simulink model "All_64_Coils"  (R2021b)
settings: Solver=FixedStepAuto  StartTime=-3000e-6  StopTime=3000e-6  FixedStep=25e-6  RelTol=1e-3
(part 2 of 70)

== system All_64_Coils/Coil-51 ==
  x : Inport
  y : Inport  Port=2
  z : Inport  Port=3
  Current : Inport  Port=4
  Add{,1..4} (5 alike) : Sum
  Display{,1..3} (4 alike) : Display
  Inner_5_coils : Subsystem
  Outer_5_Coils : Subsystem
  Scope : Scope  NumInputPorts=4
  Scope1 : Scope
  wires:
  Outer_5_Coils/3(Output2) -> Add2/2
  Inner_5_coils/2(Output1) -> Add1/1
  Outer_5_Coils/4(Output3) -> Add3/2
  z/1 -> Outer_5_Coils/3(Input2), Inner_5_coils/3(Input2)
  Add/1 -> Scope/1, Display/1  "Fxx"
  y/1 -> Outer_5_Coils/2(Input1), Inner_5_coils/2(Input1)
  Inner_5_coils/4(Output3) -> Add3/1
  Inner_5_coils/1(Output) -> Add/1
  Add3/1 -> Scope/4, Display3/1, Add4/2  "Fyz"
  Add1/1 -> Scope/2, Display1/1, Add4/1  "Fxz"
  Outer_5_Coils/1(Output) -> Add/2
  x/1 -> Outer_5_Coils/1(Input), Inner_5_coils/1(Input)
  Add2/1 -> Scope/3, Display2/1  "Fyy"
  Outer_5_Coils/2(Output1) -> Add1/2
  Current/1 -> Outer_5_Coils/4(Input3), Inner_5_coils/4(Input3)
  Inner_5_coils/3(Output2) -> Add2/1
  Add4/1 -> Scope1/1  "Total_Force"

== system All_64_Coils/Coil-52 ==
  x : Inport
  y : Inport  Port=2
  z : Inport  Port=3
  Current : Inport  Port=4
  Add{,1..4} (5 alike) : Sum
  Display{,1..3} (4 alike) : Display
  Inner_5_coils : Subsystem
  Outer_5_Coils : Subsystem
  Scope : Scope  NumInputPorts=4
  Scope1 : Scope
  wires:
  Add4/1 -> Scope1/1  "Total_Force"
  Inner_5_coils/3(Output2) -> Add2/1
  Current/1 -> Inner_5_coils/4(Input3), Outer_5_Coils/4(Input3)
  Outer_5_Coils/2(Output1) -> Add1/2
  Add2/1 -> Display2/1, Scope/3  "Fyy"
  x/1 -> Inner_5_coils/1(Input), Outer_5_Coils/1(Input)
  Outer_5_Coils/1(Output) -> Add/2
  Add1/1 -> Add4/1, Display1/1, Scope/2  "Fxz"
  Add3/1 -> Add4/2, Display3/1, Scope/4  "Fyz"
  Inner_5_coils/1(Output) -> Add/1
  Inner_5_coils/4(Output3) -> Add3/1
  y/1 -> Inner_5_coils/2(Input1), Outer_5_Coils/2(Input1)
  Add/1 -> Display/1, Scope/1  "Fxx"
  z/1 -> Inner_5_coils/3(Input2), Outer_5_Coils/3(Input2)
  Outer_5_Coils/4(Output3) -> Add3/2
  Inner_5_coils/2(Output1) -> Add1/1
  Outer_5_Coils/3(Output2) -> Add2/2

== system All_64_Coils/Coil-53 ==
  x : Inport
  y : Inport  Port=2
  z : Inport  Port=3
  Current : Inport  Port=4
  Add{,1..4} (5 alike) : Sum
  Display{,1..3} (4 alike) : Display
  Inner_5_coils : Subsystem
  Outer_5_Coils : Subsystem
  Scope : Scope  NumInputPorts=4
  Scope1 : Scope
  wires:
  Outer_5_Coils/3(Output2) -> Add2/2
  Inner_5_coils/2(Output1) -> Add1/1
  Outer_5_Coils/4(Output3) -> Add3/2
  z/1 -> Outer_5_Coils/3(Input2), Inner_5_coils/3(Input2)
  Add/1 -> Scope/1, Display/1  "Fxx"
  y/1 -> Outer_5_Coils/2(Input1), Inner_5_coils/2(Input1)
  Inner_5_coils/4(Output3) -> Add3/1
  Inner_5_coils/1(Output) -> Add/1
  Add3/1 -> Scope/4, Display3/1, Add4/2  "Fyz"
  Add1/1 -> Scope/2, Display1/1, Add4/1  "Fxz"
  Outer_5_Coils/1(Output) -> Add/2
  x/1 -> Outer_5_Coils/1(Input), Inner_5_coils/1(Input)
  Add2/1 -> Scope/3, Display2/1  "Fyy"
  Outer_5_Coils/2(Output1) -> Add1/2
  Current/1 -> Outer_5_Coils/4(Input3), Inner_5_coils/4(Input3)
  Inner_5_coils/3(Output2) -> Add2/1
  Add4/1 -> Scope1/1  "Total_Force"

== system All_64_Coils/Coil-54 ==
  x : Inport
  y : Inport  Port=2
  z : Inport  Port=3
  Current : Inport  Port=4
  Add{,1..4} (5 alike) : Sum
  Display{,1..3} (4 alike) : Display
  Inner_5_coils : Subsystem
  Outer_5_Coils : Subsystem
  Scope : Scope  NumInputPorts=4
  Scope1 : Scope
  wires:
  Add4/1 -> Scope1/1  "Total_Force"
  Inner_5_coils/3(Output2) -> Add2/1
  Current/1 -> Inner_5_coils/4(Input3), Outer_5_Coils/4(Input3)
  Outer_5_Coils/2(Output1) -> Add1/2
  Add2/1 -> Display2/1, Scope/3  "Fyy"
  x/1 -> Inner_5_coils/1(Input), Outer_5_Coils/1(Input)
  Outer_5_Coils/1(Output) -> Add/2
  Add1/1 -> Add4/1, Display1/1, Scope/2  "Fxz"
  Add3/1 -> Add4/2, Display3/1, Scope/4  "Fyz"
  Inner_5_coils/1(Output) -> Add/1
  Inner_5_coils/4(Output3) -> Add3/1
  y/1 -> Inner_5_coils/2(Input1), Outer_5_Coils/2(Input1)
  Add/1 -> Display/1, Scope/1  "Fxx"
  z/1 -> Inner_5_coils/3(Input2), Outer_5_Coils/3(Input2)
  Outer_5_Coils/4(Output3) -> Add3/2
  Inner_5_coils/2(Output1) -> Add1/1
  Outer_5_Coils/3(Output2) -> Add2/2

== system All_64_Coils/Coil-55 ==
  x : Inport
  y : Inport  Port=2
  z : Inport  Port=3
  Current : Inport  Port=4
  Add{,1..4} (5 alike) : Sum
  Display{,1..3} (4 alike) : Display
  Inner_5_coils : Subsystem
  Outer_5_Coils : Subsystem
  Scope : Scope  NumInputPorts=4
  Scope1 : Scope
  wires:
  Add4/1 -> Scope1/1  "Total_Force"
  Inner_5_coils/3(Output2) -> Add2/1
  Current/1 -> Inner_5_coils/4(Input3), Outer_5_Coils/4(Input3)
  Outer_5_Coils/2(Output1) -> Add1/2
  Add2/1 -> Display2/1, Scope/3  "Fyy"
  x/1 -> Inner_5_coils/1(Input), Outer_5_Coils/1(Input)
  Outer_5_Coils/1(Output) -> Add/2
  Add1/1 -> Add4/1, Display1/1, Scope/2  "Fxz"
  Add3/1 -> Add4/2, Display3/1, Scope/4  "Fyz"
  Inner_5_coils/1(Output) -> Add/1
  Inner_5_coils/4(Output3) -> Add3/1
  y/1 -> Inner_5_coils/2(Input1), Outer_5_Coils/2(Input1)
  Add/1 -> Display/1, Scope/1  "Fxx"
  z/1 -> Inner_5_coils/3(Input2), Outer_5_Coils/3(Input2)
  Outer_5_Coils/4(Output3) -> Add3/2
  Inner_5_coils/2(Output1) -> Add1/1
  Outer_5_Coils/3(Output2) -> Add2/2

== system All_64_Coils/Coil-56 ==
  x : Inport
  y : Inport  Port=2
  z : Inport  Port=3
  Current : Inport  Port=4
  Add{,1..4} (5 alike) : Sum
  Display{,1..3} (4 alike) : Display
  Inner_5_coils : Subsystem
  Outer_5_Coils : Subsystem
  Scope : Scope  NumInputPorts=4
  Scope1 : Scope
  wires:
  Add4/1 -> Scope1/1  "Total_Force"
  Inner_5_coils/3(Output2) -> Add2/1
  Current/1 -> Inner_5_coils/4(Input3), Outer_5_Coils/4(Input3)
  Outer_5_Coils/2(Output1) -> Add1/2
  Add2/1 -> Display2/1, Scope/3  "Fyy"
  x/1 -> Inner_5_coils/1(Input), Outer_5_Coils/1(Input)
  Outer_5_Coils/1(Output) -> Add/2
  Add1/1 -> Add4/1, Display1/1, Scope/2  "Fxz"
  Add3/1 -> Add4/2, Display3/1, Scope/4  "Fyz"
  Inner_5_coils/1(Output) -> Add/1
  Inner_5_coils/4(Output3) -> Add3/1
  y/1 -> Inner_5_coils/2(Input1), Outer_5_Coils/2(Input1)
  Add/1 -> Display/1, Scope/1  "Fxx"
  z/1 -> Inner_5_coils/3(Input2), Outer_5_Coils/3(Input2)
  Outer_5_Coils/4(Output3) -> Add3/2
  Inner_5_coils/2(Output1) -> Add1/1
  Outer_5_Coils/3(Output2) -> Add2/2

== system All_64_Coils/Coil-57 ==
  x : Inport
  y : Inport  Port=2
  z : Inport  Port=3
  Current : Inport  Port=4
  Add{,1..4} (5 alike) : Sum
  Display{,1..3} (4 alike) : Display
  Inner_5_coils : Subsystem
  Outer_5_Coils : Subsystem
  Scope : Scope  NumInputPorts=4
  Scope1 : Scope
  wires:
  Outer_5_Coils/3(Output2) -> Add2/2
  Inner_5_coils/2(Output1) -> Add1/1
  Outer_5_Coils/4(Output3) -> Add3/2
  z/1 -> Outer_5_Coils/3(Input2), Inner_5_coils/3(Input2)
  Add/1 -> Scope/1, Display/1  "Fxx"
  y/1 -> Outer_5_Coils/2(Input1), Inner_5_coils/2(Input1)
  Inner_5_coils/4(Output3) -> Add3/1
  Inner_5_coils/1(Output) -> Add/1
  Add3/1 -> Scope/4, Display3/1, Add4/2  "Fyz"
  Add1/1 -> Scope/2, Display1/1, Add4/1  "Fxz"
  Outer_5_Coils/1(Output) -> Add/2
  x/1 -> Outer_5_Coils/1(Input), Inner_5_coils/1(Input)
  Add2/1 -> Scope/3, Display2/1  "Fyy"
  Outer_5_Coils/2(Output1) -> Add1/2
  Current/1 -> Outer_5_Coils/4(Input3), Inner_5_coils/4(Input3)
  Inner_5_coils/3(Output2) -> Add2/1
  Add4/1 -> Scope1/1  "Total_Force"

== system All_64_Coils/Coil-58 ==
  x : Inport
  y : Inport  Port=2
  z : Inport  Port=3
  Current : Inport  Port=4
  Add{,1..4} (5 alike) : Sum
  Display{,1..3} (4 alike) : Display
  Inner_5_coils : Subsystem
  Outer_5_Coils : Subsystem
  Scope : Scope  NumInputPorts=4
  Scope1 : Scope
  wires:
  Outer_5_Coils/3(Output2) -> Add2/2
  Inner_5_coils/2(Output1) -> Add1/1
  Outer_5_Coils/4(Output3) -> Add3/2
  z/1 -> Outer_5_Coils/3(Input2), Inner_5_coils/3(Input2)
  Add/1 -> Scope/1, Display/1  "Fxx"
  y/1 -> Outer_5_Coils/2(Input1), Inner_5_coils/2(Input1)
  Inner_5_coils/4(Output3) -> Add3/1
  Inner_5_coils/1(Output) -> Add/1
  Add3/1 -> Scope/4, Display3/1, Add4/2  "Fyz"
  Add1/1 -> Scope/2, Display1/1, Add4/1  "Fxz"
  Outer_5_Coils/1(Output) -> Add/2
  x/1 -> Outer_5_Coils/1(Input), Inner_5_coils/1(Input)
  Add2/1 -> Scope/3, Display2/1  "Fyy"
  Outer_5_Coils/2(Output1) -> Add1/2
  Current/1 -> Outer_5_Coils/4(Input3), Inner_5_coils/4(Input3)
  Inner_5_coils/3(Output2) -> Add2/1
  Add4/1 -> Scope1/1  "Total_Force"

== system All_64_Coils/Coil-59 ==
  x : Inport
  y : Inport  Port=2
  z : Inport  Port=3
  Current : Inport  Port=4
  Add{,1..4} (5 alike) : Sum
  Display{,1..3} (4 alike) : Display
  Inner_5_coils : Subsystem
  Outer_5_Coils : Subsystem
  Scope : Scope  NumInputPorts=4
  Scope1 : Scope
  wires:
  Outer_5_Coils/3(Output2) -> Add2/2
  Inner_5_coils/2(Output1) -> Add1/1
  Outer_5_Coils/4(Output3) -> Add3/2
  z/1 -> Outer_5_Coils/3(Input2), Inner_5_coils/3(Input2)
  Add/1 -> Scope/1, Display/1  "Fxx"
  y/1 -> Outer_5_Coils/2(Input1), Inner_5_coils/2(Input1)
  Inner_5_coils/4(Output3) -> Add3/1
  Inner_5_coils/1(Output) -> Add/1
  Add3/1 -> Scope/4, Display3/1, Add4/2  "Fyz"
  Add1/1 -> Scope/2, Display1/1, Add4/1  "Fxz"
  Outer_5_Coils/1(Output) -> Add/2
  x/1 -> Outer_5_Coils/1(Input), Inner_5_coils/1(Input)
  Add2/1 -> Scope/3, Display2/1  "Fyy"
  Outer_5_Coils/2(Output1) -> Add1/2
  Current/1 -> Outer_5_Coils/4(Input3), Inner_5_coils/4(Input3)
  Inner_5_coils/3(Output2) -> Add2/1
  Add4/1 -> Scope1/1  "Total_Force"

== system All_64_Coils/Coil-6 ==
  x : Inport
  y : Inport  Port=2
  z : Inport  Port=3
  Current : Inport  Port=4
  Add{,1..4} (5 alike) : Sum
  Display{,1..3} (4 alike) : Display
  Inner_5_coils : Subsystem
  Outer_5_Coils : Subsystem
  Scope : Scope  NumInputPorts=4
  Scope1 : Scope
  wires:
  Add4/1 -> Scope1/1  "Total_Force"
  Inner_5_coils/3(Output2) -> Add2/1
  Current/1 -> Inner_5_coils/4(Input3), Outer_5_Coils/4(Input3)
  Outer_5_Coils/2(Output1) -> Add1/2
  Add2/1 -> Display2/1, Scope/3  "Fyy"
  x/1 -> Inner_5_coils/1(Input), Outer_5_Coils/1(Input)
  Outer_5_Coils/1(Output) -> Add/2
  Add1/1 -> Add4/1, Display1/1, Scope/2  "Fxz"
  Add3/1 -> Add4/2, Display3/1, Scope/4  "Fyz"
  Inner_5_coils/1(Output) -> Add/1
  Inner_5_coils/4(Output3) -> Add3/1
  y/1 -> Inner_5_coils/2(Input1), Outer_5_Coils/2(Input1)
  Add/1 -> Display/1, Scope/1  "Fxx"
  z/1 -> Inner_5_coils/3(Input2), Outer_5_Coils/3(Input2)
  Outer_5_Coils/4(Output3) -> Add3/2
  Inner_5_coils/2(Output1) -> Add1/1
  Outer_5_Coils/3(Output2) -> Add2/2

== system All_64_Coils/Coil-60 ==
  x : Inport
  y : Inport  Port=2
  z : Inport  Port=3
  Current : Inport  Port=4
  Add{,1..4} (5 alike) : Sum
  Display{,1..3} (4 alike) : Display
  Inner_5_coils : Subsystem
  Outer_5_Coils : Subsystem
  Scope : Scope  NumInputPorts=4
  Scope1 : Scope
  wires:
  Add4/1 -> Scope1/1  "Total_Force"
  Inner_5_coils/3(Output2) -> Add2/1
  Current/1 -> Inner_5_coils/4(Input3), Outer_5_Coils/4(Input3)
  Outer_5_Coils/2(Output1) -> Add1/2
  Add2/1 -> Display2/1, Scope/3  "Fyy"
  x/1 -> Inner_5_coils/1(Input), Outer_5_Coils/1(Input)
  Outer_5_Coils/1(Output) -> Add/2
  Add1/1 -> Add4/1, Display1/1, Scope/2  "Fxz"
  Add3/1 -> Add4/2, Display3/1, Scope/4  "Fyz"
  Inner_5_coils/1(Output) -> Add/1
  Inner_5_coils/4(Output3) -> Add3/1
  y/1 -> Inner_5_coils/2(Input1), Outer_5_Coils/2(Input1)
  Add/1 -> Display/1, Scope/1  "Fxx"
  z/1 -> Inner_5_coils/3(Input2), Outer_5_Coils/3(Input2)
  Outer_5_Coils/4(Output3) -> Add3/2
  Inner_5_coils/2(Output1) -> Add1/1
  Outer_5_Coils/3(Output2) -> Add2/2

== system All_64_Coils/Coil-61 ==
  x : Inport
  y : Inport  Port=2
  z : Inport  Port=3
  Current : Inport  Port=4
  Add{,1..4} (5 alike) : Sum
  Display{,1..3} (4 alike) : Display
  Inner_5_coils : Subsystem
  Outer_5_Coils : Subsystem
  Scope : Scope  NumInputPorts=4
  Scope1 : Scope
  wires:
  Outer_5_Coils/3(Output2) -> Add2/2
  Inner_5_coils/2(Output1) -> Add1/1
  Outer_5_Coils/4(Output3) -> Add3/2
  z/1 -> Outer_5_Coils/3(Input2), Inner_5_coils/3(Input2)
  Add/1 -> Scope/1, Display/1  "Fxx"
  y/1 -> Outer_5_Coils/2(Input1), Inner_5_coils/2(Input1)
  Inner_5_coils/4(Output3) -> Add3/1
  Inner_5_coils/1(Output) -> Add/1
  Add3/1 -> Scope/4, Display3/1, Add4/2  "Fyz"
  Add1/1 -> Scope/2, Display1/1, Add4/1  "Fxz"
  Outer_5_Coils/1(Output) -> Add/2
  x/1 -> Outer_5_Coils/1(Input), Inner_5_coils/1(Input)
  Add2/1 -> Scope/3, Display2/1  "Fyy"
  Outer_5_Coils/2(Output1) -> Add1/2
  Current/1 -> Outer_5_Coils/4(Input3), Inner_5_coils/4(Input3)
  Inner_5_coils/3(Output2) -> Add2/1
  Add4/1 -> Scope1/1  "Total_Force"

== system All_64_Coils/Coil-62 ==
  x : Inport
  y : Inport  Port=2
  z : Inport  Port=3
  Current : Inport  Port=4
  Add{,1..4} (5 alike) : Sum
  Display{,1..3} (4 alike) : Display
  Inner_5_coils : Subsystem
  Outer_5_Coils : Subsystem
  Scope : Scope  NumInputPorts=4
  Scope1 : Scope
  wires:
  Outer_5_Coils/3(Output2) -> Add2/2
  Inner_5_coils/2(Output1) -> Add1/1
  Outer_5_Coils/4(Output3) -> Add3/2
  z/1 -> Outer_5_Coils/3(Input2), Inner_5_coils/3(Input2)
  Add/1 -> Scope/1, Display/1  "Fxx"
  y/1 -> Outer_5_Coils/2(Input1), Inner_5_coils/2(Input1)
  Inner_5_coils/4(Output3) -> Add3/1
  Inner_5_coils/1(Output) -> Add/1
  Add3/1 -> Scope/4, Display3/1, Add4/2  "Fyz"
  Add1/1 -> Scope/2, Display1/1, Add4/1  "Fxz"
  Outer_5_Coils/1(Output) -> Add/2
  x/1 -> Outer_5_Coils/1(Input), Inner_5_coils/1(Input)
  Add2/1 -> Scope/3, Display2/1  "Fyy"
  Outer_5_Coils/2(Output1) -> Add1/2
  Current/1 -> Outer_5_Coils/4(Input3), Inner_5_coils/4(Input3)
  Inner_5_coils/3(Output2) -> Add2/1
  Add4/1 -> Scope1/1  "Total_Force"

== system All_64_Coils/Coil-63 ==
  x : Inport
  y : Inport  Port=2
  z : Inport  Port=3
  Current : Inport  Port=4
  Add{,1..4} (5 alike) : Sum
  Display{,1..3} (4 alike) : Display
  Inner_5_coils : Subsystem
  Outer_5_Coils : Subsystem
  Scope : Scope  NumInputPorts=4
  Scope1 : Scope
  wires:
  Add4/1 -> Scope1/1  "Total_Force"
  Inner_5_coils/3(Output2) -> Add2/1
  Current/1 -> Inner_5_coils/4(Input3), Outer_5_Coils/4(Input3)
  Outer_5_Coils/2(Output1) -> Add1/2
  Add2/1 -> Display2/1, Scope/3  "Fyy"
  x/1 -> Inner_5_coils/1(Input), Outer_5_Coils/1(Input)
  Outer_5_Coils/1(Output) -> Add/2
  Add1/1 -> Add4/1, Display1/1, Scope/2  "Fxz"
  Add3/1 -> Add4/2, Display3/1, Scope/4  "Fyz"
  Inner_5_coils/1(Output) -> Add/1
  Inner_5_coils/4(Output3) -> Add3/1
  y/1 -> Inner_5_coils/2(Input1), Outer_5_Coils/2(Input1)
  Add/1 -> Display/1, Scope/1  "Fxx"
  z/1 -> Inner_5_coils/3(Input2), Outer_5_Coils/3(Input2)
  Outer_5_Coils/4(Output3) -> Add3/2
  Inner_5_coils/2(Output1) -> Add1/1
  Outer_5_Coils/3(Output2) -> Add2/2

== system All_64_Coils/Coil-64 ==
  x : Inport
  y : Inport  Port=2
  z : Inport  Port=3
  Current : Inport  Port=4
  Add{,1..4} (5 alike) : Sum
  Display{,1..3} (4 alike) : Display
  Inner_5_coils : Subsystem
  Outer_5_Coils : Subsystem
  Scope : Scope  NumInputPorts=4
  Scope1 : Scope
  wires:
  Add4/1 -> Scope1/1  "Total_Force"
  Inner_5_coils/3(Output2) -> Add2/1
  Current/1 -> Inner_5_coils/4(Input3), Outer_5_Coils/4(Input3)
  Outer_5_Coils/2(Output1) -> Add1/2
  Add2/1 -> Display2/1, Scope/3  "Fyy"
  x/1 -> Inner_5_coils/1(Input), Outer_5_Coils/1(Input)
  Outer_5_Coils/1(Output) -> Add/2
  Add1/1 -> Add4/1, Display1/1, Scope/2  "Fxz"
  Add3/1 -> Add4/2, Display3/1, Scope/4  "Fyz"
  Inner_5_coils/1(Output) -> Add/1
  Inner_5_coils/4(Output3) -> Add3/1
  y/1 -> Inner_5_coils/2(Input1), Outer_5_Coils/2(Input1)
  Add/1 -> Display/1, Scope/1  "Fxx"
  z/1 -> Inner_5_coils/3(Input2), Outer_5_Coils/3(Input2)
  Outer_5_Coils/4(Output3) -> Add3/2
  Inner_5_coils/2(Output1) -> Add1/1
  Outer_5_Coils/3(Output2) -> Add2/2

== system All_64_Coils/Coil-7 ==
  x : Inport
  y : Inport  Port=2
  z : Inport  Port=3
  Current : Inport  Port=4
  Add{,1..4} (5 alike) : Sum
  Display{,1..3} (4 alike) : Display
  Inner_5_coils : Subsystem
  Outer_5_Coils : Subsystem
  Scope : Scope  NumInputPorts=4
  Scope1 : Scope
  wires:
  Add4/1 -> Scope1/1  "Total_Force"
  Inner_5_coils/3(Output2) -> Add2/1
  Current/1 -> Inner_5_coils/4(Input3), Outer_5_Coils/4(Input3)
  Outer_5_Coils/2(Output1) -> Add1/2
  Add2/1 -> Display2/1, Scope/3  "Fyy"
  x/1 -> Inner_5_coils/1(Input), Outer_5_Coils/1(Input)
  Outer_5_Coils/1(Output) -> Add/2
  Add1/1 -> Add4/1, Display1/1, Scope/2  "Fxz"
  Add3/1 -> Add4/2, Display3/1, Scope/4  "Fyz"
  Inner_5_coils/1(Output) -> Add/1
  Inner_5_coils/4(Output3) -> Add3/1
  y/1 -> Inner_5_coils/2(Input1), Outer_5_Coils/2(Input1)
  Add/1 -> Display/1, Scope/1  "Fxx"
  z/1 -> Inner_5_coils/3(Input2), Outer_5_Coils/3(Input2)
  Outer_5_Coils/4(Output3) -> Add3/2
  Inner_5_coils/2(Output1) -> Add1/1
  Outer_5_Coils/3(Output2) -> Add2/2

== system All_64_Coils/Coil-8 ==
  x : Inport
  y : Inport  Port=2
  z : Inport  Port=3
  Current : Inport  Port=4
  Add{,1..4} (5 alike) : Sum
  Display{,1..3} (4 alike) : Display
  Inner_5_coils : Subsystem
  Outer_5_Coils : Subsystem
  Scope : Scope  NumInputPorts=4
  Scope1 : Scope
  wires:
  Outer_5_Coils/3(Output2) -> Add2/2
  Inner_5_coils/2(Output1) -> Add1/1
  Outer_5_Coils/4(Output3) -> Add3/2
  z/1 -> Outer_5_Coils/3(Input2), Inner_5_coils/3(Input2)
  Add/1 -> Scope/1, Display/1  "Fxx"
  y/1 -> Outer_5_Coils/2(Input1), Inner_5_coils/2(Input1)
  Inner_5_coils/4(Output3) -> Add3/1
  Inner_5_coils/1(Output) -> Add/1
  Add3/1 -> Scope/4, Display3/1, Add4/2  "Fyz"
  Add1/1 -> Scope/2, Display1/1, Add4/1  "Fxz"
  Outer_5_Coils/1(Output) -> Add/2
  x/1 -> Outer_5_Coils/1(Input), Inner_5_coils/1(Input)
  Add2/1 -> Scope/3, Display2/1  "Fyy"
  Outer_5_Coils/2(Output1) -> Add1/2
  Current/1 -> Outer_5_Coils/4(Input3), Inner_5_coils/4(Input3)
  Inner_5_coils/3(Output2) -> Add2/1
  Add4/1 -> Scope1/1  "Total_Force"

== system All_64_Coils/Coil-9 ==
  x : Inport
  y : Inport  Port=2
  z : Inport  Port=3
  Current : Inport  Port=4
  Add{,1..4} (5 alike) : Sum
  Display{,1..3} (4 alike) : Display
  Inner_5_coils : Subsystem
  Outer_5_Coils : Subsystem
  Scope : Scope  NumInputPorts=4
  Scope1 : Scope
  wires:
  Outer_5_Coils/3(Output2) -> Add2/2
  Inner_5_coils/2(Output1) -> Add1/1
  Outer_5_Coils/4(Output3) -> Add3/2
  z/1 -> Outer_5_Coils/3(Input2), Inner_5_coils/3(Input2)
  Add/1 -> Scope/1, Display/1  "Fxx"
  y/1 -> Outer_5_Coils/2(Input1), Inner_5_coils/2(Input1)
  Inner_5_coils/4(Output3) -> Add3/1
  Inner_5_coils/1(Output) -> Add/1
  Add3/1 -> Scope/4, Display3/1, Add4/2  "Fyz"
  Add1/1 -> Scope/2, Display1/1, Add4/1  "Fxz"
  Outer_5_Coils/1(Output) -> Add/2
  x/1 -> Outer_5_Coils/1(Input), Inner_5_coils/1(Input)
  Add2/1 -> Scope/3, Display2/1  "Fyy"
  Outer_5_Coils/2(Output1) -> Add1/2
  Current/1 -> Outer_5_Coils/4(Input3), Inner_5_coils/4(Input3)
  Inner_5_coils/3(Output2) -> Add2/1
  Add4/1 -> Scope1/1  "Total_Force"

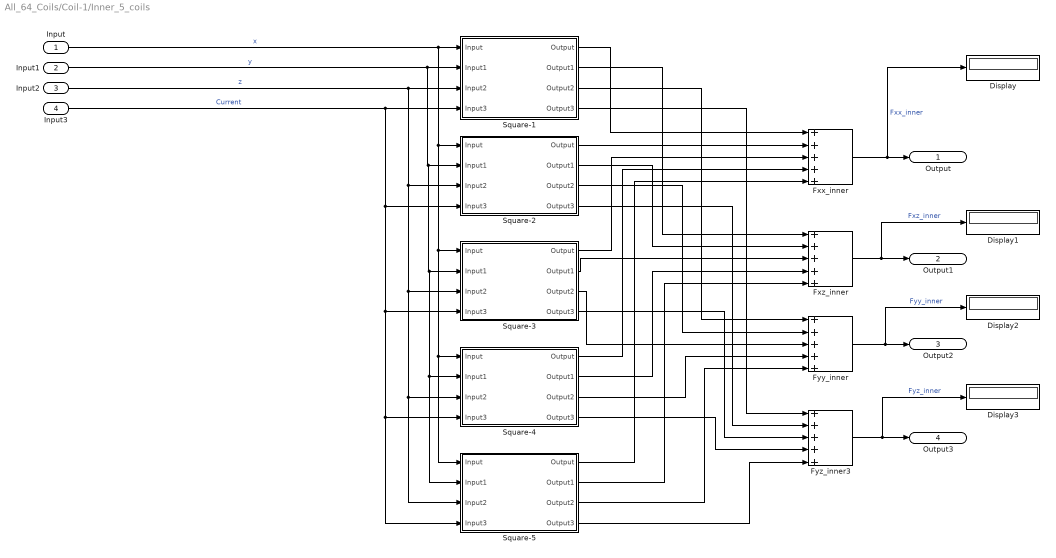
== system All_64_Coils/Coil-1/Inner_5_coils ==
  Input : Inport
  Input1 : Inport  Port=2
  Input2 : Inport  Port=3
  Input3 : Inport  Port=4
  Display{,1..3} (4 alike) : Display
  Fxx_inner : Sum  Inputs=+++++
  Fxz_inner : Sum  Inputs=+++++
  Fyy_inner : Sum  Inputs=+++++
  Fyz_inner3 : Sum  Inputs=+++++
  Square-1 : Subsystem
  Square-2 : Subsystem
  Square-3 : Subsystem
  Square-4 : Subsystem
  Square-5 : Subsystem
  Output : Outport
  Output1 : Outport  Port=2
  Output2 : Outport  Port=3
  Output3 : Outport  Port=4
  wires:
  Input/1 -> Square-5/1(Input), Square-4/1(Input), Square-3/1(Input), Square-2/1(Input), Square-1/1(Input)  "x"
  Input3/1 -> Square-2/4(Input3), Square-3/4(Input3), Square-4/4(Input3), Square-5/4(Input3), Square-1/4(Input3)  "Current"
  Input1/1 -> Square-5/2(Input1), Square-4/2(Input1), Square-3/2(Input1), Square-2/2(Input1), Square-1/2(Input1)  "y"
  Input2/1 -> Square-1/3(Input2), Square-3/3(Input2), Square-4/3(Input2), Square-5/3(Input2), Square-2/3(Input2)  "z"
  Square-1/1(Output) -> Fxx_inner/1
  Square-2/1(Output) -> Fxx_inner/2
  Square-3/1(Output) -> Fxx_inner/3
  Square-4/1(Output) -> Fxx_inner/4
  Square-5/1(Output) -> Fxx_inner/5
  Fxx_inner/1 -> Display/1, Output/1  "Fxx_inner"
  Fxz_inner/1 -> Display1/1, Output1/1  "Fxz_inner"
  Fyy_inner/1 -> Display2/1, Output2/1  "Fyy_inner"
  Fyz_inner3/1 -> Display3/1, Output3/1  "Fyz_inner"
  Square-1/2(Output1) -> Fxz_inner/1
  Square-2/2(Output1) -> Fxz_inner/2
  Square-3/2(Output1) -> Fxz_inner/3
  Square-4/2(Output1) -> Fxz_inner/4
  Square-5/2(Output1) -> Fxz_inner/5
  Square-1/3(Output2) -> Fyy_inner/1
  Square-2/3(Output2) -> Fyy_inner/2
  Square-3/3(Output2) -> Fyy_inner/3
  Square-4/3(Output2) -> Fyy_inner/4
  Square-5/3(Output2) -> Fyy_inner/5
  Square-1/4(Output3) -> Fyz_inner3/1
  Square-2/4(Output3) -> Fyz_inner3/2
  Square-3/4(Output3) -> Fyz_inner3/3
  Square-4/4(Output3) -> Fyz_inner3/4
  Square-5/4(Output3) -> Fyz_inner3/5

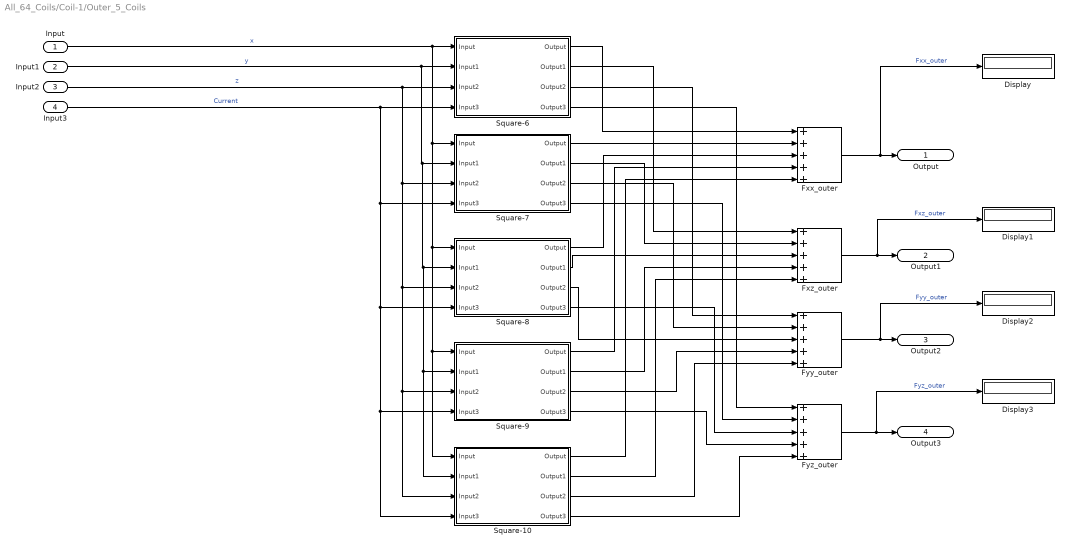
== system All_64_Coils/Coil-1/Outer_5_Coils ==
  Input : Inport
  Input1 : Inport  Port=2
  Input2 : Inport  Port=3
  Input3 : Inport  Port=4
  Display{,1..3} (4 alike) : Display
  Fxx_outer : Sum  Inputs=+++++
  Fxz_outer : Sum  Inputs=+++++
  Fyy_outer : Sum  Inputs=+++++
  Fyz_outer : Sum  Inputs=+++++
  Square-10 : Subsystem
  Square-6 : Subsystem
  Square-7 : Subsystem
  Square-8 : Subsystem
  Square-9 : Subsystem
  Output : Outport
  Output1 : Outport  Port=2
  Output2 : Outport  Port=3
  Output3 : Outport  Port=4
  wires:
  Square-10/4(Output3) -> Fyz_outer/5
  Square-9/4(Output3) -> Fyz_outer/4
  Square-8/4(Output3) -> Fyz_outer/3
  Square-7/4(Output3) -> Fyz_outer/2
  Square-6/4(Output3) -> Fyz_outer/1
  Square-10/3(Output2) -> Fyy_outer/5
  Square-9/3(Output2) -> Fyy_outer/4
  Square-8/3(Output2) -> Fyy_outer/3
  Square-7/3(Output2) -> Fyy_outer/2
  Square-6/3(Output2) -> Fyy_outer/1
  Square-10/2(Output1) -> Fxz_outer/5
  Square-9/2(Output1) -> Fxz_outer/4
  Square-8/2(Output1) -> Fxz_outer/3
  Square-7/2(Output1) -> Fxz_outer/2
  Square-6/2(Output1) -> Fxz_outer/1
  Fyz_outer/1 -> Display3/1, Output3/1  "Fyz_outer"
  Fyy_outer/1 -> Display2/1, Output2/1  "Fyy_outer"
  Fxz_outer/1 -> Display1/1, Output1/1  "Fxz_outer"
  Fxx_outer/1 -> Display/1, Output/1  "Fxx_outer"
  Square-10/1(Output) -> Fxx_outer/5
  Square-9/1(Output) -> Fxx_outer/4
  Square-8/1(Output) -> Fxx_outer/3
  Square-7/1(Output) -> Fxx_outer/2
  Square-6/1(Output) -> Fxx_outer/1
  Input2/1 -> Square-7/3(Input2), Square-10/3(Input2), Square-9/3(Input2), Square-8/3(Input2), Square-6/3(Input2)  "z"
  Input1/1 -> Square-6/2(Input1), Square-7/2(Input1), Square-8/2(Input1), Square-9/2(Input1), Square-10/2(Input1)  "y"
  Input3/1 -> Square-6/4(Input3), Square-10/4(Input3), Square-9/4(Input3), Square-8/4(Input3), Square-7/4(Input3)  "Current"
  Input/1 -> Square-6/1(Input), Square-7/1(Input), Square-8/1(Input), Square-9/1(Input), Square-10/1(Input)  "x"

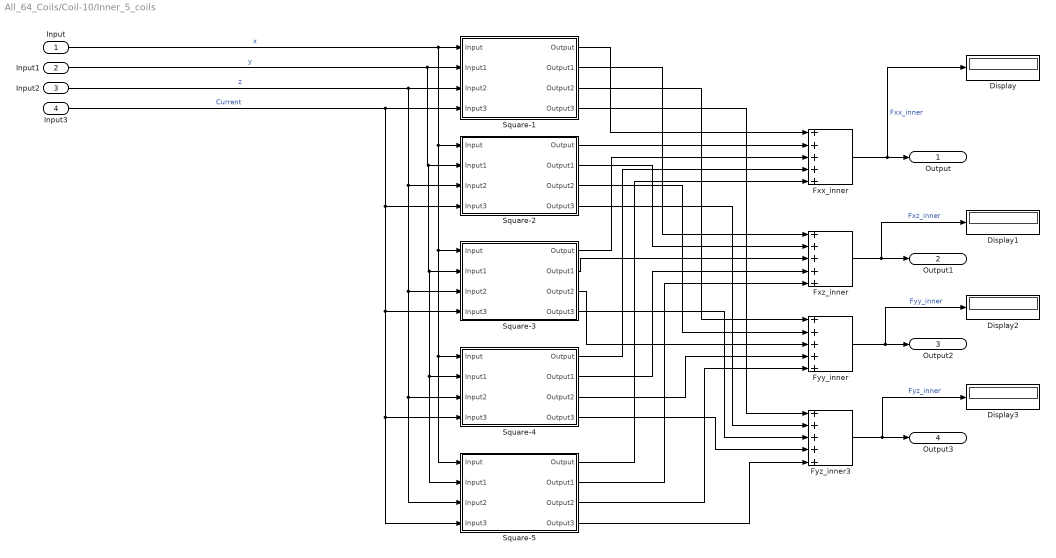
== system All_64_Coils/Coil-10/Inner_5_coils ==
  Input : Inport
  Input1 : Inport  Port=2
  Input2 : Inport  Port=3
  Input3 : Inport  Port=4
  Display{,1..3} (4 alike) : Display
  Fxx_inner : Sum  Inputs=+++++
  Fxz_inner : Sum  Inputs=+++++
  Fyy_inner : Sum  Inputs=+++++
  Fyz_inner3 : Sum  Inputs=+++++
  Square-1 : Subsystem
  Square-2 : Subsystem
  Square-3 : Subsystem
  Square-4 : Subsystem
  Square-5 : Subsystem
  Output : Outport
  Output1 : Outport  Port=2
  Output2 : Outport  Port=3
  Output3 : Outport  Port=4
  wires:
  Input/1 -> Square-5/1(Input), Square-4/1(Input), Square-3/1(Input), Square-2/1(Input), Square-1/1(Input)  "x"
  Input3/1 -> Square-2/4(Input3), Square-3/4(Input3), Square-4/4(Input3), Square-5/4(Input3), Square-1/4(Input3)  "Current"
  Input1/1 -> Square-5/2(Input1), Square-4/2(Input1), Square-3/2(Input1), Square-2/2(Input1), Square-1/2(Input1)  "y"
  Input2/1 -> Square-1/3(Input2), Square-3/3(Input2), Square-4/3(Input2), Square-5/3(Input2), Square-2/3(Input2)  "z"
  Square-1/1(Output) -> Fxx_inner/1
  Square-2/1(Output) -> Fxx_inner/2
  Square-3/1(Output) -> Fxx_inner/3
  Square-4/1(Output) -> Fxx_inner/4
  Square-5/1(Output) -> Fxx_inner/5
  Fxx_inner/1 -> Display/1, Output/1  "Fxx_inner"
  Fxz_inner/1 -> Display1/1, Output1/1  "Fxz_inner"
  Fyy_inner/1 -> Display2/1, Output2/1  "Fyy_inner"
  Fyz_inner3/1 -> Display3/1, Output3/1  "Fyz_inner"
  Square-1/2(Output1) -> Fxz_inner/1
  Square-2/2(Output1) -> Fxz_inner/2
  Square-3/2(Output1) -> Fxz_inner/3
  Square-4/2(Output1) -> Fxz_inner/4
  Square-5/2(Output1) -> Fxz_inner/5
  Square-1/3(Output2) -> Fyy_inner/1
  Square-2/3(Output2) -> Fyy_inner/2
  Square-3/3(Output2) -> Fyy_inner/3
  Square-4/3(Output2) -> Fyy_inner/4
  Square-5/3(Output2) -> Fyy_inner/5
  Square-1/4(Output3) -> Fyz_inner3/1
  Square-2/4(Output3) -> Fyz_inner3/2
  Square-3/4(Output3) -> Fyz_inner3/3
  Square-4/4(Output3) -> Fyz_inner3/4
  Square-5/4(Output3) -> Fyz_inner3/5
